simulink model "ofdm_txrx_alamouti"  (Simulink 6.3)
settings: Solver=ode45  StartTime=0.0  StopTime=45000  RelTol=1e-3
InitFcn:
  | ofdm_rx_alamouti_init
StartFcn:
  | ofdm_rx_alamouti_init
values from neighbouring files (symbols this model uses):
  CPLength = 16    [ofdm_tx_alamouti_init.m]
  CRCPolynomial = hex2dec('04c11db7')    [calcTxCRC.m]    (also in ofdm_tx_alamouti_init.m)
  hi = zeros(1,length(cci))    [ofdm_rx_alamouti_init.m]
  hr = zeros(1,length(ccr))    [ofdm_rx_alamouti_init.m]
  max_CP_length = 16    [ofdm_tx_alamouti_init.m]
  max_num_baseRateSymbols = 31    [ofdm_tx_alamouti_init.m]
  max_num_subcarriers = 64    [ofdm_tx_alamouti_init.m]
  max_num_trainingSymbols = 15    [ofdm_tx_alamouti_init.m]
  max_OFDM_symbols = 2047    [ofdm_tx_alamouti_init.m]
  modConstellation_qam16 = 0.75*[1 3 -1 -3]./3    [ofdm_tx_alamouti_init.m]
  modConstellation_qam256 = 0.9375*[3 1 5 7 11 9 13 15 -3 -1 -5 -7 -11 -9 -13 -15]./15    [ofdm_tx_alamouti_init.m]
  modConstellation_qam64 = 0.875*[3 1 5 7 -3 -1 -5 -7]./7    [ofdm_tx_alamouti_init.m]
  modConstellation_qpsk = [1 -1]./sqrt(2)    [ofdm_tx_alamouti_init.m]
  numBaseRateSymbols = 2    [ofdm_tx_alamouti_init.m]
  numFullRateSymbols = numFullRateSymbols + mod(numFullRateSymbols, 2)    [ofdm_tx_alamouti_init.m]
  numSubcarriers = 64    [ofdm_tx_alamouti_init.m]
  numTrainingSymbols = 4    [ofdm_tx_alamouti_init.m]
  pkt_crossCorr_thresh = 0.7    [ofdm_rx_alamouti_init.m]
  pkt_energy_thresh = 0    [ofdm_rx_alamouti_init.m]
  pkt_numPayloadBytes = 24+4+20+20+500    (= 568)    [ofdm_tx_alamouti_init.m]
  pktTiming_count_payloadStart = pktTiming_count_lsStart + pktTiming_ls_num*pktTiming_ls_length    [ofdm_rx_init_packetTimingControl.m]
  preamble = 6*[0 shortsyms_10 longsyms_2]    [ofdm_tx_alamouti_init.m]
  preamble_I = real(preamble)    [ofdm_tx_alamouti_init.m]
  preamble_Q = imag(preamble)    [ofdm_tx_alamouti_init.m]
  RAM_init_size = 4096    [ofdm_tx_alamouti_init.m]
  rx_longCorrCountLoad = 251-16    (= 235)    [ofdm_rx_alamouti_init.m]
  rx_longCorrThresh = 3000    [ofdm_rx_alamouti_init.m]
  RxDataScrambling_Seq = [40 198 78 63 82 173 102 245 48 111 172 115 147 230 216 93 72 65 62 2 205 242 122 90 128 83 105 97 73 10 5 252]    [ofdm_tx_alamouti_init.m]
  simOnly_numSamples = length(preamble)+( (numSubcarriers+CPLength)*(numTrainingSymbols + numBaseRateSymbols + numFullRateSymbols) )    [ofdm_tx_alamouti_init.m]
  train = [train train]    [ofdm_tx_alamouti_init.m]
  TxDataScrambling_Seq = [40 198 78 63 82 173 102 245 48 111 172 115 147 230 216 93 72 65 62 2 205 242 122 90 128 83 105 97 73 10 5 252]    [ofdm_tx_alamouti_init.m]
undefined symbols (not defined in the file or in its folder): CRC_table_gen, gcbh, interpFilter_4, xlBlockLoadCallback
libraries: xbsIndex_r4 x4373, dspmlti4 x4, dspsigops x4, commchan3 x2, simulink x2, commrflib2 x2, commsink2 x2, dspsrcs4 x1
(part 5 of 39)

== system ofdm_txrx_alamouti/Registers/Tx Slices & Gotos ==
  note: LSB
  note: 0x1
  note: 0x2
  note: 0x4
  note: 0x8
  note: 0xF0
  note: 0x100
  note: 0xFFFF0000
  note: 0x200
  note: 0x400
  note: 0x800
  32b : Inport
  Slice1 : xbsIndex_r4/Slice  nbits=1  boolean_output=off  mode=Lower Bit Location + Width  bit1=0  base1=MSB of Input  bit0=10  base0=LSB of Input  dbl_ovrd=off  block_type=slice
      settings: infoedit="Extracts a given range of bits from each input sample and presents it at the output.  The output type is ordinarily unsigned with binary point at zero, but can be Boolean when the slice is one bit wide.<P><P>Hardware notes: In hardware this block costs nothing."  block_version=VER_STRING_GOES_HERE
  Slice14 : xbsIndex_r4/Slice  nbits=1  boolean_output=on  mode=Lower Bit Location + Width  bit1=0  base1=MSB of Input  bit0=0  base0=LSB of Input  dbl_ovrd=off  block_type=slice
      settings: infoedit="Extracts a given range of bits from each input sample and presents it at the output.  The output type is ordinarily unsigned with binary point at zero, but can be Boolean when the slice is one bit wide.<P><P>Hardware notes: In hardware this block costs nothing."  block_version=VER_STRING_GOES_HERE
  Slice18 : xbsIndex_r4/Slice  nbits=1  boolean_output=on  mode=Lower Bit Location + Width  bit1=0  base1=MSB of Input  bit0=1  base0=LSB of Input  dbl_ovrd=off  block_type=slice
      settings: infoedit="Extracts a given range of bits from each input sample and presents it at the output.  The output type is ordinarily unsigned with binary point at zero, but can be Boolean when the slice is one bit wide.<P><P>Hardware notes: In hardware this block costs nothing."  block_version=VER_STRING_GOES_HERE
  Slice19 : xbsIndex_r4/Slice  nbits=1  boolean_output=on  mode=Lower Bit Location + Width  bit1=0  base1=MSB of Input  bit0=2  base0=LSB of Input  dbl_ovrd=off  block_type=slice
      settings: infoedit="Extracts a given range of bits from each input sample and presents it at the output.  The output type is ordinarily unsigned with binary point at zero, but can be Boolean when the slice is one bit wide.<P><P>Hardware notes: In hardware this block costs nothing."  block_version=VER_STRING_GOES_HERE
  Slice2 : xbsIndex_r4/Slice  nbits=1  boolean_output=off  mode=Lower Bit Location + Width  bit1=0  base1=MSB of Input  bit0=11  base0=LSB of Input  dbl_ovrd=off  block_type=slice
      settings: infoedit="Extracts a given range of bits from each input sample and presents it at the output.  The output type is ordinarily unsigned with binary point at zero, but can be Boolean when the slice is one bit wide.<P><P>Hardware notes: In hardware this block costs nothing."  block_version=VER_STRING_GOES_HERE
  Slice20 : xbsIndex_r4/Slice  nbits=1  boolean_output=on  mode=Lower Bit Location + Width  bit1=0  base1=MSB of Input  bit0=3  base0=LSB of Input  dbl_ovrd=off  block_type=slice
      settings: infoedit="Extracts a given range of bits from each input sample and presents it at the output.  The output type is ordinarily unsigned with binary point at zero, but can be Boolean when the slice is one bit wide.<P><P>Hardware notes: In hardware this block costs nothing."  block_version=VER_STRING_GOES_HERE
  Slice21 : xbsIndex_r4/Slice  nbits=4  boolean_output=off  mode=Lower Bit Location + Width  bit1=0  base1=MSB of Input  bit0=4  base0=LSB of Input  dbl_ovrd=off  block_type=slice
      settings: infoedit="Extracts a given range of bits from each input sample and presents it at the output.  The output type is ordinarily unsigned with binary point at zero, but can be Boolean when the slice is one bit wide.<P><P>Hardware notes: In hardware this block costs nothing."  block_version=VER_STRING_GOES_HERE
  Slice27 : xbsIndex_r4/Slice  nbits=16  boolean_output=off  mode=Lower Bit Location + Width  bit1=0  base1=MSB of Input  bit0=16  base0=LSB of Input  dbl_ovrd=off  block_type=slice
      settings: infoedit="Extracts a given range of bits from each input sample and presents it at the output.  The output type is ordinarily unsigned with binary point at zero, but can be Boolean when the slice is one bit wide.<P><P>Hardware notes: In hardware this block costs nothing."  block_version=VER_STRING_GOES_HERE
  Slice28 : xbsIndex_r4/Slice  nbits=1  boolean_output=off  mode=Lower Bit Location + Width  bit1=0  base1=MSB of Input  bit0=8  base0=LSB of Input  dbl_ovrd=off  block_type=slice
      settings: infoedit="Extracts a given range of bits from each input sample and presents it at the output.  The output type is ordinarily unsigned with binary point at zero, but can be Boolean when the slice is one bit wide.<P><P>Hardware notes: In hardware this block costs nothing."  block_version=VER_STRING_GOES_HERE
  Slice29 : xbsIndex_r4/Slice  nbits=1  boolean_output=off  mode=Lower Bit Location + Width  bit1=0  base1=MSB of Input  bit0=9  base0=LSB of Input  dbl_ovrd=off  block_type=slice
      settings: infoedit="Extracts a given range of bits from each input sample and presents it at the output.  The output type is ordinarily unsigned with binary point at zero, but can be Boolean when the slice is one bit wide.<P><P>Hardware notes: In hardware this block costs nothing."  block_version=VER_STRING_GOES_HERE
  tag scope: TxReg_2ndTxAntB=global, TxReg_2ndTxDelay=global, TxReg_2ndTxEn=global, TxReg_AntBPreambleShift=global, TxReg_DisableAntBPreamble=global, TxReg_EnableTxPreCFO=global, TxReg_PilotScrambling=global, TxReg_RelayTxEn=global
  wires:
  32b/1 -> Slice1/1, Slice27/1, Slice2/1, Slice29/1, Slice28/1, Slice21/1, Slice20/1, Slice19/1, Slice18/1, Slice14/1
  Slice19/1 -> [TxReg_DisableAntBPreamble]
  Slice20/1 -> [TxReg_PilotScrambling]
  Slice21/1 -> [TxReg_AntBPreambleShift]
  Slice27/1 -> [TxReg_2ndTxDelay]
  Slice28/1 -> [TxReg_2ndTxEn]
  Slice29/1 -> [TxReg_2ndTxAntB]
  Slice1/1 -> [TxReg_EnableTxPreCFO]
  Slice2/1 -> [TxReg_RelayTxEn]

== system ofdm_txrx_alamouti/OFDM Rx Alamouti/ADCs & Ant Selection/Antenna Selection ==
  AntA_I : Inport
  AntA_Q : Inport  Port=2
  AntB_I : Inport  Port=3
  AntB_Q : Inport  Port=4
  PktDetReset : Inport  Port=5
  Ant Sel Logic : Subsystem
  AntA_Gains : Subsystem
  AntB_Gains : Subsystem
  Concat2 : xbsIndex_r4/Concat  num_inputs=3  dbl_ovrd=off  block_type=concat
      settings: block_version=VER_STRING_GOES_HERE
  Constant6 : xbsIndex_r4/Constant  arith_type=Unsigned  const=0  n_bits=1  bin_pt=0  explicit_period=off  period=1  equ=P=C  opselect=C  inp2=PCIN>>17  opr=+  inp1=P  carry=CIN  dbl_ovrd=off  block_type=constant
      settings: block_version=VER_STRING_GOES_HERE
  Mux : xbsIndex_r4/Mux  inputs=2  en=off  latency=1  precision=Full  arith_type=Unsigned  n_bits=16  bin_pt=14  quantization=Truncate  overflow=Wrap  dbl_ovrd=off  block_type=mux
      settings: block_version=8.2.02
  Mux1 : xbsIndex_r4/Mux  inputs=2  en=off  latency=1  precision=Full  arith_type=Unsigned  n_bits=16  bin_pt=14  quantization=Truncate  overflow=Wrap  dbl_ovrd=off  block_type=mux
      settings: block_version=8.2.02
  Posedge2 : Subsystem
  Register4 : S-Function  LoadFcn=xlBlockLoadCallback(gcbh);  FunctionName=sysgen  Parameters=ptable_  mask(Xilinx Register Block){init=0, rst_sgadvanced=, rst=off, en_sgadvanced=, en=off, dbl_ovrd_sgadvanced=, dbl_ovrd=off, xl_use_area_sgadvanced=, xl_use_area=off, xl_area=[7 14 0 0 0 0 0], has_advanced_control=0, sggui_pos=-1,-1,-1,-1, block_type=register, block_version=VER_STRING_GOES_HERE, sg_icon_stat=30,28,1,1,white,blue,0,ac6b57db, sg_mask_display=|, sg_list_contents=, sg_blockgui_xml=|}
      mask sg_mask_display=|
      | fprintf('','COMMENT: begin icon graphics ');
      | patch([0 30 30 0 ],[0 0 28 28 ],[0.77 0.82 0.91]);
      | patch([8 3 10 3 8 15 17 19 27 21 15 11 19 11 15 21 27 19 17 15 8 ],[3 8 15 22 27 27 25 27 27 21 27 23 15 7 3 9 3 3 5 3 3 ],[0.98 0.96 0.92]);
      | plot([0 0 30 30 0 ],[0 28 28 0 0 ]);
      | fprintf('','COMMENT: end icon graphics');
      |
      | fprintf('','COMMENT: begin icon text ');
      | color('black');port_label('input',1,'d');
      | color('black');port_label('output',1,'q');
      | color('black');disp('\bf{z^{-1}}','texmode','on');
      | fprintf('','COMMENT: end icon text');
      mask sg_blockgui_xml=|
      | <!--   *  Copyright (c) 2005, Xilinx, Inc.  All Rights Reserved.           --><!--   *  Reproduction or reuse, in any form, without the explicit written --><!--   *  consent of Xilinx, Inc., is strictly prohibited.                 --><sysgenblock block_type="register" simulinkname="Register" >
      |  <initialization file="xlregister_init.m" />
      |  <icon width="60" bg_color="blue" height="56" wmark_color="white" />
      |  <dll entry_point="Register_config" name="Sysgen" />
      |  <handlers enablement="xlmagicenablement" />
      |  <libraries>
      |   <library name="xbsIndex" />
      |   <library name="xbsBasic" />
      |   <library name="xbsControl" />
      |   <library name="xbsMemory" />
      |  </libraries>
      |  <blockgui label="Xilinx Register" >
      |   <tabpane>
      |    <tab name="basictab" label="Basic" >
      |     <editbox ctype="Double" evaluate="true" name="init" label="Initial value" default="0" />
      |     <etch label="Optional Ports" >
      |      <checkbox ctype="Int" evaluate="true" name="rst" label="Provide synchronous reset port" default="off" />
      |      <checkbox ctype="Int" evaluate="true" name="en" label="Provide enable port" default="off" />
      |     </etch>
      |    </tab>
      |    <tab name="advancedtab" label="Advanced" >
      |     <etch name="simetch" label="Simulation" >
      |      <checkbox ctype="Int" evaluate="true" name="dbl_ovrd" label="Override with doubles" default="off" />
      |     </etch>
      |    </tab>
      |    <tab name="impltab" label="Implementation" >
      |     <etch label="FPGA Area Estimation" >
      |      <checkbox name="xl_use_area" label="Define FPGA area for resource estimation" default="off" />
      |      <editbox top_label="true" name="xl_area" label="FPGA area [slices, FFs, BRAMs, LUTs, IOBs, emb. mults, TBUFs]" default="[0,0,0,0,0,0,0]" />
      |     </etch>
      |    </tab>
      |   </tabpane>
      |  </blockgui>
      | </sysgenblock>
      mask prompts: init "Initial value"; rst_sgadvanced ""; rst "Provide synchronous reset port"; en_sgadvanced ""; en "Provide enable port"; dbl_ovrd_sgadvanced ""; dbl_ovrd "Override with doubles"; xl_use_area_sgadvanced ""; xl_use_area "Define FPGA area for resource estimation"; xl_area "FPGA area [slices, FFs, BRAMs, LUTs, IOBs, emb. mults, TBUFs]"; has_advanced_control ""; sggui_pos ""; block_type ""; block_version ""; sg_icon_stat ""; sg_mask_display ""; sg_list_contents ""; sg_blockgui_xml ""
      mask help: Xilinx Register
      mask init:
      | try
      |   tmp_gcb = gcb;
      |   tmp_gcbh = gcbh;
      |   xlMungeMaskParams;
      |   block_config = 'Sysgen:Register_config';
      |   serialized_declarations = '{''dbl_ovrd''=>''Int'',''en''=>''Int'',''init''=>''Double'',''rst''=>''Int''}';
      |   xlregister_init();
      |   ptable_ = xlblockprep(get_param(tmp_gcb, 'MaskWSVariables'));
      | catch
      |   global xl_report_errors;
      |   if(~isempty(xl_report_errors) && xl_report_errors)
      |     e = regexprep(lasterr, '\n', '\nError: ');
      |     disp(['Error: While running MaskInit code on block ' tmp_gcb ': ' e]);
      |     error(e);
      |   end
      | end
      settings: CopyFcn=xlBlockMoveCallback(gcbh);  DeleteFcn=xlDestroyGui(gcbh);  ModelCloseFcn=xlDestroyGui(gcbh);  PreSaveFcn=xlBlockPreSaveCallback(gcbh);  PostSaveFcn=xlBlockPostSaveCallback(gcbh);  DestroyFcn=xlDestroyGui(gcbh);  OpenFcn=xlOpenGui(gcbh, @xlmagicenablement, -1)  CloseFcn=xlDestroyGui(gcbh);  MoveFcn=xlBlockMoveCallback(gcbh);
      mask Help: eval('xlWeb(xlhtmldoclink(''Register''))');
      mask Display:
      | fprintf('','COMMENT: begin icon graphics ');
      | patch([0 30 30 0 ],[0 0 28 28 ],[0.77 0.82 0.91]);
      | patch([8 3 10 3 8 15 17 19 27 21 15 11 19 11 15 21 27 19 17 15 8 ],[3 8 15 22 27 27 25 27 27 21 27 23 15 7 3 9 3 3 5 3 3 ],[0.98 0.96 0.92]);
      | plot([0 0 30 30 0 ],[0 28 28 0 0 ]);
      | fprintf('','COMMENT: end icon graphics');
      | 
      | fprintf('','COMMENT: begin icon text ');
      | color('black');port_label('input',1,'d');
      | color('black');port_label('output',1,'q');
      | color('black');disp('\bf{z^{-1}}','texmode','on');
      | fprintf('','COMMENT: end icon text');
  Relational : xbsIndex_r4/Relational  mode=a>b  en=off  latency=0  dbl_ovrd=off  block_type=relational
      settings: block_version=8.2.02
  Rx_Gains : xbsIndex_r4/To Register  shared_memory_name='Rx_Gains'  init=0  ownership=Locally owned and initialized  explicit_data_type=off  arith_type="Signed  (2's comp)"  n_bits=16  bin_pt=14  dbl_ovrd=off  block_type=toreg
      settings: AttributesFormatString=<< %<shared_memory_name> >>  infoedit="Register block that writes data to a shared memory register.  Delay of one sample period."  block_version=VER_STRING_GOES_HERE
  Rx_debug_antSel : xbsIndex_r4/Gateway Out  hdl_port=on  timing_constraint=None  locs_specified=off  LOCs={}  block_type=gatewayout
      settings: infoedit="Gateway out block.  Converts Xilinx fixed point inputs into ouputs of type Simulink integer, double, or fixed point.<P><P>Hardware notes:  In hardware these blocks become top level output ports or are discarded, depending on how they are configured."  xl_area=[0 0 0 0 1 0 0]  block_version=VER_STRING_GOES_HERE
  Terminator : Terminator
  Up Sample : xbsIndex_r4/Up Sample  sample_ratio=4  copy_samples=on  dbl_ovrd=off  block_type=usamp
      settings: infoedit="Up samples input data.  Inserted values  can be zeros or copies of the most recent input sample.<P><P>Hardware notes: No hardware is needed if inserted values are copies of the input sample; otherwise, a mux and single bit flip-flop are used."  block_version=VER_STRING_GOES_HERE
  Up Sample1 : xbsIndex_r4/Up Sample  sample_ratio=4  copy_samples=on  dbl_ovrd=off  block_type=usamp
      settings: infoedit="Up samples input data.  Inserted values  can be zeros or copies of the most recent input sample.<P><P>Hardware notes: No hardware is needed if inserted values are copies of the input sample; otherwise, a mux and single bit flip-flop are used."  block_version=VER_STRING_GOES_HERE
  Up Sample2 : xbsIndex_r4/Up Sample  sample_ratio=4  copy_samples=on  dbl_ovrd=off  block_type=usamp
      settings: infoedit="Up samples input data.  Inserted values  can be zeros or copies of the most recent input sample.<P><P>Hardware notes: No hardware is needed if inserted values are copies of the input sample; otherwise, a mux and single bit flip-flop are used."  block_version=VER_STRING_GOES_HERE
  I : Outport
  Q : Outport  Port=2
  wires:
  AntB_I/1 -> Mux/3
  AntB_Q/1 -> Mux1/3
  AntA_I/1 -> Mux/2
  AntA_Q/1 -> Mux1/2
  Mux/1 -> I/1
  Mux1/1 -> Q/1
  PktDetReset/1 -> Up Sample1/1, AntB_Gains/1(Rst), AntA_Gains/1(Rst)
  AntA_Gains/2(GainVec) -> Relational/1, Concat2/3
  AntB_Gains/2(GainVec) -> Relational/2, Concat2/1
  Relational/1 -> Ant Sel Logic/1(G(B) < G(A))
  Ant Sel Logic/1(AntSel) -> Mux/1, Mux1/1, Up Sample2/1
  Up Sample2/1 -> Register4/1
  AntB_Gains/1(Vout) -> Ant Sel Logic/2(B_Vin)
  Concat2/1 -> Up Sample/1
  Up Sample/1 -> Rx_Gains/1
  Posedge2/1(Q) -> Rx_Gains/2
  Constant6/1 -> Concat2/2
  Register4/1 -> Rx_debug_antSel/1
  Up Sample1/1 -> Posedge2/1(D)
  Rx_Gains/1 -> Terminator/1

== system ofdm_txrx_alamouti/OFDM Rx Alamouti/Coarse Freq Correction1/A*B ==
  Re[A] : Inport
  Im[A] : Inport  Port=2
  Im[B] : Inport  Port=3
  Re[B] : Inport  Port=4
  Add1 : xbsIndex_r4/AddSub  mode=Addition  use_carryin=off  use_carryout=off  en=off  latency=0  precision=User Defined  arith_type="Signed  (2's comp)"  n_bits=17  bin_pt=15  quantization=Truncate  overflow=Flag as error  dbl_ovrd=off  use_behavioral_HDL=on  pipelined=off  use_rpm=on  block_type=addsub
      settings: xl_area=[9 0 0 16 0 0 0]  block_version=VER_STRING_GOES_HERE
  Counter : xbsIndex_r4/Counter  Orientation=down  cnt_type=Free Running  cnt_to=4  operation=Up  start_count=0  cnt_by_val=1  arith_type=Unsigned  n_bits=2  bin_pt=0  load_pin=off  rst=off  en=off  explicit_period=on  period=1  dbl_ovrd=off  use_behavioral_HDL=off  use_rpm=on  block_type=counter
      settings: xl_area=[3 3 0 4 0 0 0]  block_version=VER_STRING_GOES_HERE
  Delay1 : xbsIndex_r4/Delay  en=off  latency=1  dbl_ovrd=off  reg_retiming=off  block_type=delay
      settings: infoedit=Hardware notes: A delay line is a chain, each link of which is an SRL16 followed by a flip-flop. If register retiming is enabled, the delay line is a chain of flip-flops.  xl_area=[8 16 0 0 0 0 0]  block_version=VER_STRING_GOES_HERE
  Delay2 : xbsIndex_r4/Delay  en=off  latency=2  dbl_ovrd=off  reg_retiming=off  block_type=delay
      settings: infoedit=Hardware notes: A delay line is a chain, each link of which is an SRL16 followed by a flip-flop. If register retiming is enabled, the delay line is a chain of flip-flops.  xl_area=[9 17 0 17 0 0 0]  block_version=VER_STRING_GOES_HERE
  Down Sample3 : xbsIndex_r4/Down Sample  sample_ratio=4  sample_phase=First Value of Frame  en=off  latency=1  dbl_ovrd=off  block_type=dsamp
      settings: xl_area=[9 17 0 0 0 0 0]  block_version=VER_STRING_GOES_HERE
  Down Sample4 : xbsIndex_r4/Down Sample  sample_ratio=4  sample_phase=First Value of Frame  en=off  latency=1  dbl_ovrd=off  block_type=dsamp
      settings: xl_area=[9 17 0 0 0 0 0]  block_version=VER_STRING_GOES_HERE
  Mult4 : xbsIndex_r4/Mult  precision=User Defined  arith_type="Signed  (2's comp)"  n_bits=17  bin_pt=16  quantization=Truncate  overflow=Flag as error  en=off  latency=1  dbl_ovrd=off  use_behavioral_HDL=off  use_embedded=on  opt=Speed  optimum_pipeline=off  use_rpm=on  placement_style=Rectangular shape  pipeline=off  block_type=mult
      settings: infoedit=Hardware notes: To use the internal pipeline stage of the dedicated multiplier you must select 'Pipeline for maximum performance'.  xl_area=[14 27 0 27 0 1 0]  block_version=VER_STRING_GOES_HERE
  Mux : xbsIndex_r4/Mux  inputs=4  en=off  latency=0  precision=Full  arith_type="Signed  (2's comp)"  n_bits=16  bin_pt=13  quantization=Truncate  overflow=Wrap  dbl_ovrd=off  block_type=mux
      settings: xl_area=[14 0 0 28 0 0 0]  block_version=VER_STRING_GOES_HERE
  Mux1 : xbsIndex_r4/Mux  inputs=4  en=off  latency=0  precision=Full  arith_type="Signed  (2's comp)"  n_bits=16  bin_pt=13  quantization=Truncate  overflow=Wrap  dbl_ovrd=off  block_type=mux
      settings: xl_area=[14 0 0 28 0 0 0]  block_version=VER_STRING_GOES_HERE
  Negate : xbsIndex_r4/Negate  precision=Full  arith_type="Signed  (2's comp)"  n_bits=14  bin_pt=13  quantization=Truncate  overflow=Flag as error  en=off  latency=0  dbl_ovrd=off  block_type=negate
      settings: xl_area=[7 0 0 14 0 0 0]  block_version=VER_STRING_GOES_HERE
  Slice : xbsIndex_r4/Slice  nbits=2  boolean_output=off  mode=Lower Bit Location + Width  bit1=0  base1=MSB of Input  bit0=0  base0=LSB of Input  dbl_ovrd=off  block_type=slice
      settings: infoedit="Extracts a given range of bits from each input sample and presents it at the output.  The output type is ordinarily unsigned with binary point at zero, but can be Boolean when the slice is one bit wide.<P><P>Hardware notes: In hardware this block costs nothing."  block_version=VER_STRING_GOES_HERE
  Up Sample{1..4} (4 alike) : xbsIndex_r4/Up Sample  sample_ratio=4  copy_samples=on  dbl_ovrd=off  block_type=usamp
      settings: infoedit="Up samples input data.  Inserted values  can be zeros or copies of the most recent input sample.<P><P>Hardware notes: No hardware is needed if inserted values are copies of the input sample; otherwise, a mux and single bit flip-flop are used."  xl_area=[0 0 0 0 0 0 0]  block_version=VER_STRING_GOES_HERE
  Re : Outport
  Im1 : Outport  Port=2
  wires:
  Negate/1 -> Mux1/3
  Add1/1 -> Down Sample4/1, Delay2/1
  Down Sample3/1 -> Re/1
  Down Sample4/1 -> Im1/1
  Delay2/1 -> Down Sample3/1
  Mux/1 -> Mult4/2
  Mux1/1 -> Mult4/1
  Im[B]/1 -> Up Sample4/1
  Im[A]/1 -> Up Sample1/1
  Re[B]/1 -> Up Sample2/1
  Re[A]/1 -> Up Sample3/1
  Up Sample3/1 -> Mux1/2, Mux1/4
  Up Sample2/1 -> Mux/5, Mux/2
  Up Sample4/1 -> Mux/3, Mux/4
  Up Sample1/1 -> Negate/1, Mux1/5
  Mult4/1 -> Add1/1, Delay1/1
  Delay1/1 -> Add1/2
  Counter/1 -> Slice/1
  Slice/1 -> Mux1/1, Mux/1

== system ofdm_txrx_alamouti/OFDM Rx Alamouti/Coarse Freq Correction1/Coarse Freq Offset Calc Combo ==
  I In : Inport
  Q In : Inport  Port=2
  Reset : Inport  Port=3
  PktDet : Inport  Port=4
  Accum : xbsIndex_r4/Accumulator  operation=Add  n_bits=7+13  overflow=Flag as error  scale=1  rst=on  hasbypass=on  en=on  dbl_ovrd=off  use_behavioral_HDL=on  block_type=accum
      settings: infoedit="Adder or subtractor-based accumulator.   Output type and binary point position match the input.<P><P>Hardware notes: When \"Reinitialize with input 'b' on reset\" is selected, the accumulator is forced to run at the system rate even if the input 'b' is running at a slower rate."  xl_area=[17 33 0 32 0 0 0]  block_version=VER_STRING_GOES_HERE
  Accum Control : Subsystem
  Arctan2 : Subsystem
  Coarse Freq Scope1 : Scope  NumInputPorts=5
      settings: TimeRange=3500  YMin=-3.5~0~0~0~0  YMax=3.5~1~75~0.00035~0.000275  SaveName=ScopeData39
  Coarse Freq Scope4 : Scope  NumInputPorts=5
      settings: TimeRange=3500  YMin=-5~-0.0001~0~0~0  YMax=12.5~0.00035~0.00035~1~1  SaveName=ScopeData43
  Convert : xbsIndex_r4/Convert  arith_type=Unsigned  n_bits=32  bin_pt=32  quantization=Truncate  overflow=Wrap  latency=0  dbl_ovrd=off  pipeline=off  block_type=convert
      settings: xl_area=[0 0 0 0 0 0 0]  block_version=VER_STRING_GOES_HERE
  Convert1 : xbsIndex_r4/Convert  arith_type=Unsigned  n_bits=32  bin_pt=32  quantization=Truncate  overflow=Wrap  latency=0  dbl_ovrd=off  pipeline=off  block_type=convert
      settings: xl_area=[0 0 0 0 0 0 0]  block_version=VER_STRING_GOES_HERE
  Counter : xbsIndex_r4/Counter  cnt_type=Free Running  cnt_to=Inf  operation=Up  start_count=0  cnt_by_val=1  arith_type=Unsigned  n_bits=7  bin_pt=0  load_pin=off  rst=on  en=on  explicit_period=off  period=1  dbl_ovrd=off  use_behavioral_HDL=off  use_rpm=on  block_type=counter
      settings: xl_area=[6 11 0 12 0 0 0]  block_version=VER_STRING_GOES_HERE
  Delay1 : xbsIndex_r4/Delay  en=off  latency=4  dbl_ovrd=off  reg_retiming=off  block_type=delay
      settings: infoedit=Hardware notes: A delay line is a chain, each link of which is an SRL16 followed by a flip-flop. If register retiming is enabled, the delay line is a chain of flip-flops.  block_version=VER_STRING_GOES_HERE
  Delay4 : xbsIndex_r4/Delay  en=off  latency=4  dbl_ovrd=off  reg_retiming=off  block_type=delay
      settings: infoedit=Hardware notes: A delay line is a chain, each link of which is an SRL16 followed by a flip-flop. If register retiming is enabled, the delay line is a chain of flip-flops.  block_version=VER_STRING_GOES_HERE
  Delay5 : xbsIndex_r4/Delay  en=off  latency=1  dbl_ovrd=off  reg_retiming=off  block_type=delay
      settings: infoedit=Hardware notes: A delay line is a chain, each link of which is an SRL16 followed by a flip-flop. If register retiming is enabled, the delay line is a chain of flip-flops.  block_version=VER_STRING_GOES_HERE
  Delay6 : xbsIndex_r4/Delay  en=off  latency=2  dbl_ovrd=off  reg_retiming=off  block_type=delay
      settings: infoedit=Hardware notes: A delay line is a chain, each link of which is an SRL16 followed by a flip-flop. If register retiming is enabled, the delay line is a chain of flip-flops.  block_version=VER_STRING_GOES_HERE
  Gateway Out{1..5,19..23} (10 alike) : xbsIndex_r4/Gateway Out  hdl_port=off  timing_constraint=None  locs_specified=off  LOCs={}  block_type=gatewayout
      settings: infoedit="Gateway out block.  Converts Xilinx fixed point inputs into ouputs of type Simulink integer, double, or fixed point.<P><P>Hardware notes:  In hardware these blocks become top level output ports or are discarded, depending on how they are configured."  xl_area=[0 0 0 0 0 0 0]  block_version=VER_STRING_GOES_HERE
  Inverter1 : xbsIndex_r4/Inverter  en=off  latency=0  dbl_ovrd=off  block_type=inv
      settings: xl_area=[0 0 0 0 0 0 0]  block_version=VER_STRING_GOES_HERE
  Inverter2 : xbsIndex_r4/Inverter  en=off  latency=0  dbl_ovrd=off  block_type=inv
      settings: xl_area=[0 0 0 0 0 0 0]  block_version=VER_STRING_GOES_HERE
  Mult1 : xbsIndex_r4/Mult  precision=User Defined  arith_type="Signed  (2's comp)"  n_bits=35  bin_pt=33  quantization="Round  (unbiased: +/- Inf)"  overflow=Flag as error  en=off  latency=2  dbl_ovrd=off  use_behavioral_HDL=off  use_embedded=on  opt=Speed  optimum_pipeline=off  use_rpm=off  placement_style=Rectangular shape  pipeline=off  block_type=mult
      settings: infoedit=Hardware notes: To use the internal pipeline stage of the dedicated multiplier you must select 'Pipeline for maximum performance'.  xl_area=[52 69 0 102 0 2 0]  block_version=VER_STRING_GOES_HERE
  Mux2 : xbsIndex_r4/Mux  inputs=2  en=off  latency=0  precision=Full  arith_type="Signed  (2's comp)"  n_bits=8  bin_pt=2  quantization=Truncate  overflow=Wrap  dbl_ovrd=off  block_type=mux
      settings: xl_area=[0, 0, 0, 0, 0, 0, 0]  block_version=VER_STRING_GOES_HERE
  Phase Noise Tracker1 : Subsystem
  PhaseDiff : Subsystem
  Posedge : Subsystem
  Posedge1 : Subsystem
  Register1 : xbsIndex_r4/Register  init=0  rst=on  en=on  dbl_ovrd=off  block_type=register
      settings: block_version=8.2.01
  Register4 : xbsIndex_r4/Register  init=0  rst=on  en=on  dbl_ovrd=off  block_type=register
      settings: block_version=8.2.01
  inv 2pi : xbsIndex_r4/Constant  arith_type=Unsigned  const=1/(2*pi*64*72)  n_bits=24  bin_pt=24  explicit_period=off  period=1  equ=P=C  opselect=C  inp2=PCIN>>17  opr=+  inp1=P  carry=CIN  dbl_ovrd=off  block_type=constant
      settings: block_version=VER_STRING_GOES_HERE
  Phase Inc : Outport
  wires:
  PktDet/1 -> Accum Control/1(En)
  Q In/1 -> Arctan2/2(Q)
  I In/1 -> Arctan2/1(I)
  Accum Control/1(" En") -> Delay4/1
  Gateway Out19/1 -> Coarse Freq Scope4/1
  Gateway Out20/1 -> Coarse Freq Scope4/2
  Gateway Out21/1 -> Coarse Freq Scope4/3
  Gateway Out22/1 -> Coarse Freq Scope4/4
  Gateway Out23/1 -> Coarse Freq Scope4/5
  PhaseDiff/1(diff(p0,p63)) -> Accum/1, Gateway Out1/1, Phase Noise Tracker1/1(Phase Err)
  Inverter1/1 -> Accum/2, Posedge/1(D)
  Delay4/1 -> Delay5/1
  Accum/1 -> Mult1/1, Gateway Out22/1
  inv 2pi/1 -> Mult1/2
  Mult1/1 -> Register4/1, Gateway Out21/1
  Posedge/1(Q) -> Delay6/1
  Delay6/1 -> Register4/3, Gateway Out20/1
  Register4/1 -> Gateway Out19/1, Gateway Out5/1, Convert1/1
  Reset/1 -> Accum Control/2(Reset), Register4/2, Counter/1, Phase Noise Tracker1/2(Reset), Register1/2, Gateway Out23/1
  Arctan2/1(atan) -> PhaseDiff/1(p)
  Delay5/1 -> Inverter1/1, Accum/3, Counter/2, Gateway Out2/1, Phase Noise Tracker1/3(Vin), Inverter2/1
  Counter/1 -> Gateway Out3/1, Phase Noise Tracker1/4(Samp Index)
  Gateway Out1/1 -> Coarse Freq Scope1/1
  Gateway Out2/1 -> Coarse Freq Scope1/2
  Gateway Out3/1 -> Coarse Freq Scope1/3
  Gateway Out4/1 -> Coarse Freq Scope1/4
  Gateway Out5/1 -> Coarse Freq Scope1/5
  Phase Noise Tracker1/1(theta) -> Gateway Out4/1, Register1/1
  Convert/1 -> Mux2/3
  Register1/1 -> Convert/1
  Delay1/1 -> Register1/3
  Inverter2/1 -> Posedge1/1(D)
  Posedge1/1(Q) -> Delay1/1
  Convert1/1 -> Mux2/2
  [regRx_newCoarseCFO] -> Mux2/1
  Mux2/1 -> Phase Inc/1

== system ofdm_txrx_alamouti/OFDM Rx Alamouti/Coarse Freq Correction1/DDS ==
  PhaseInc : Inport
  Rst : Inport  Port=2
  "DDS v4_0 " : xbsIndex_r4/DDS v4_0   Description=Direct Digital Synthesizer  mode=Sine and Cosine  neg_sin=on  neg_cos=off  n_bits=24  phase_increment_type=Register  phase_increment=1/10  phase_offset_type=Constant  phase_offset=0  rst=on  en=off  lut_addr_bits=10  acc_latency=One Cycle  acc_n_bits=32  phase_dithering=off  dbl_ovrd=off  explicit_period=off  period=1  mem_type=Block RAM  pipeline=off  use_rpm=off  block_type=ddsv4
      settings: block_version=VER_STRING_GOES_HERE
  Logical1 : xbsIndex_r4/Logical  logical_function=OR  inputs=2  en=off  latency=0  precision=Full  arith_type=Unsigned  n_bits=8  bin_pt=2  align_bp=on  dbl_ovrd=off  block_type=logical
      settings: xl_area=[1 0 0 1 0 0 0]  block_version=VER_STRING_GOES_HERE
  inv 2pi2 : xbsIndex_r4/Constant  arith_type=Boolean  const=1  n_bits=32  bin_pt=32  explicit_period=off  period=1  equ=P=C  opselect=C  inp2=PCIN>>17  opr=+  inp1=P  carry=CIN  dbl_ovrd=off  block_type=constant
      settings: block_version=VER_STRING_GOES_HERE
  Q : Outport
  I : Outport  Port=2
  wires:
  "DDS v4_0 "/2 -> I/1
  "DDS v4_0 "/1 -> Q/1
  Rst/1 -> Logical1/1
  inv 2pi2/1 -> "DDS v4_0 "/2
  PhaseInc/1 -> "DDS v4_0 "/1
  Logical1/1 -> "DDS v4_0 "/3
  [regRx_freqCorrBypass] -> Logical1/2

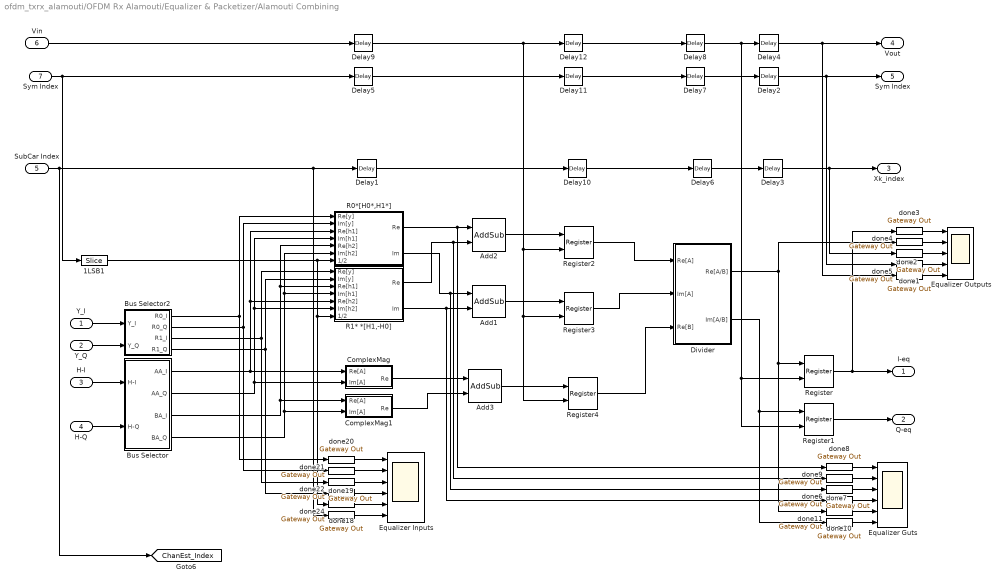
== system ofdm_txrx_alamouti/OFDM Rx Alamouti/Equalizer & Packetizer/Alamouti Combining ==
  Y_I : Inport
  Y_Q : Inport  Port=2
  H-I : Inport  Port=3
  H-Q : Inport  Port=4
  SubCar Index : Inport  Port=5
  Vin : Inport  Port=6
  Sym Index : Inport  Port=7
  1LSB1 : xbsIndex_r4/Slice  nbits=1  boolean_output=off  mode=Lower Bit Location + Width  bit1=0  base1=MSB of Input  bit0=0  base0=LSB of Input  dbl_ovrd=off  block_type=slice
      settings: infoedit="Extracts a given range of bits from each input sample and presents it at the output.  The output type is ordinarily unsigned with binary point at zero, but can be Boolean when the slice is one bit wide.<P><P>Hardware notes: In hardware this block costs nothing."  block_version=VER_STRING_GOES_HERE
  Add1 : xbsIndex_r4/AddSub  mode=Addition  use_carryin=off  use_carryout=off  en=off  latency=0  precision=Full  arith_type="Signed  (2's comp)"  n_bits=18  bin_pt=16  quantization=Truncate  overflow=Flag as error  dbl_ovrd=off  use_behavioral_HDL=on  pipelined=off  use_rpm=on  block_type=addsub
      settings: xl_area=[9 0 0 16 0 0 0]  block_version=VER_STRING_GOES_HERE
  Add2 : xbsIndex_r4/AddSub  mode=Addition  use_carryin=off  use_carryout=off  en=off  latency=0  precision=Full  arith_type="Signed  (2's comp)"  n_bits=18  bin_pt=16  quantization=Truncate  overflow=Flag as error  dbl_ovrd=off  use_behavioral_HDL=on  pipelined=off  use_rpm=on  block_type=addsub
      settings: xl_area=[9 0 0 16 0 0 0]  block_version=VER_STRING_GOES_HERE
  Add3 : xbsIndex_r4/AddSub  mode=Addition  use_carryin=off  use_carryout=off  en=off  latency=0  precision=Full  arith_type="Signed  (2's comp)"  n_bits=18  bin_pt=16  quantization=Truncate  overflow=Flag as error  dbl_ovrd=off  use_behavioral_HDL=on  pipelined=off  use_rpm=on  block_type=addsub
      settings: xl_area=[9 0 0 16 0 0 0]  block_version=VER_STRING_GOES_HERE
  Bus Selector : Subsystem
  Bus Selector2 : Subsystem
  ComplexMag : Subsystem
  ComplexMag1 : Subsystem
  Delay1 : xbsIndex_r4/Delay  en=off  latency=2  dbl_ovrd=off  reg_retiming=off  block_type=delay
      settings: infoedit=Hardware notes: A delay line is a chain, each link of which is an SRL16 followed by a flip-flop. If register retiming is enabled, the delay line is a chain of flip-flops.  xl_area=[0, 0, 0, 0, 0, 0, 0]  block_version=VER_STRING_GOES_HERE
  Delay{2..4,10..12} (6 alike) : xbsIndex_r4/Delay  en=off  latency=1  dbl_ovrd=off  reg_retiming=off  block_type=delay
      settings: infoedit=Hardware notes: A delay line is a chain, each link of which is an SRL16 followed by a flip-flop. If register retiming is enabled, the delay line is a chain of flip-flops.  xl_area=[0, 0, 0, 0, 0, 0, 0]  block_version=VER_STRING_GOES_HERE
  Delay5 : xbsIndex_r4/Delay  en=off  latency=2  dbl_ovrd=off  reg_retiming=off  block_type=delay
      settings: infoedit=Hardware notes: A delay line is a chain, each link of which is an SRL16 followed by a flip-flop. If register retiming is enabled, the delay line is a chain of flip-flops.  xl_area=[0, 0, 0, 0, 0, 0, 0]  block_version=VER_STRING_GOES_HERE
  Delay6 : xbsIndex_r4/Delay  en=off  latency=23-2  dbl_ovrd=off  reg_retiming=off  block_type=delay
      settings: infoedit=Hardware notes: A delay line is a chain, each link of which is an SRL16 followed by a flip-flop. If register retiming is enabled, the delay line is a chain of flip-flops.  xl_area=[0, 0, 0, 0, 0, 0, 0]  block_version=VER_STRING_GOES_HERE
  Delay7 : xbsIndex_r4/Delay  en=off  latency=23-2  dbl_ovrd=off  reg_retiming=off  block_type=delay
      settings: infoedit=Hardware notes: A delay line is a chain, each link of which is an SRL16 followed by a flip-flop. If register retiming is enabled, the delay line is a chain of flip-flops.  xl_area=[0, 0, 0, 0, 0, 0, 0]  block_version=VER_STRING_GOES_HERE
  Delay8 : xbsIndex_r4/Delay  en=off  latency=23-2  dbl_ovrd=off  reg_retiming=off  block_type=delay
      settings: infoedit=Hardware notes: A delay line is a chain, each link of which is an SRL16 followed by a flip-flop. If register retiming is enabled, the delay line is a chain of flip-flops.  xl_area=[0, 0, 0, 0, 0, 0, 0]  block_version=VER_STRING_GOES_HERE
  Delay9 : xbsIndex_r4/Delay  en=off  latency=2  dbl_ovrd=off  reg_retiming=off  block_type=delay
      settings: infoedit=Hardware notes: A delay line is a chain, each link of which is an SRL16 followed by a flip-flop. If register retiming is enabled, the delay line is a chain of flip-flops.  xl_area=[0, 0, 0, 0, 0, 0, 0]  block_version=VER_STRING_GOES_HERE
  Divider : Subsystem
  Equalizer Guts : Scope  NumInputPorts=6
      settings: TimeRange=4000  YMin=-0.125~-0.1~-0.125~-0.125~-1.25~-1  YMax=0.125~0.125~0.125~0.1~1.25~1.25  SaveName=ScopeData38
  Equalizer Outputs : Scope  NumInputPorts=5
      settings: TimeRange=4000  YMin=-0.075~-0.2~0~15~0  YMax=0.075~0.2~70~21~1  SaveName=ScopeData26
  Equalizer Inputs : Scope  NumInputPorts=6
      settings: TimeRange=12000  YMin=-0.5~-0.5~-0.5~-0.5~0~0  YMax=0.5~1~0.5~1~1~100  SaveName=ScopeData18
  R0*[H0*,H1*] : Subsystem
  R1* *[H1,-H0] : Subsystem
  Register{,1..4} (5 alike) : xbsIndex_r4/Register  init=0  rst=off  en=on  dbl_ovrd=off  block_type=register
      settings: block_version=VER_STRING_GOES_HERE
  done{1..11,18..22,24} (17 alike) : xbsIndex_r4/Gateway Out  hdl_port=off  timing_constraint=None  locs_specified=off  LOCs={}  block_type=gatewayout
      settings: infoedit="Gateway out block.  Converts Xilinx fixed point inputs into ouputs of type Simulink integer, double, or fixed point.<P><P>Hardware notes:  In hardware these blocks become top level output ports or are discarded, depending on how they are configured."  xl_area=[0 0 0 0 1 0 0]  block_version=VER_STRING_GOES_HERE
  I-eq : Outport
  Q-eq : Outport  Port=2
  " Xk_index" : Outport  Port=3
  Vout : Outport  Port=4
  " Sym Index" : Outport  Port=5
  tag scope: ChanEst_Index=global
  wires:
  H-I/1 -> Bus Selector/1(H-I)
  H-Q/1 -> Bus Selector/2(H-Q)
  SubCar Index/1 -> [ChanEst_Index], Delay1/1, done24/1
  done18/1 -> Equalizer Inputs/5
  done20/1 -> Equalizer Inputs/1
  done21/1 -> Equalizer Inputs/2
  done19/1 -> Equalizer Inputs/3
  done22/1 -> Equalizer Inputs/4
  done24/1 -> Equalizer Inputs/6
  done3/1 -> Equalizer Outputs/1
  done4/1 -> Equalizer Outputs/2
  done2/1 -> Equalizer Outputs/3
  Vin/1 -> Delay9/1
  done1/1 -> Equalizer Outputs/4
  Sym Index/1 -> Delay5/1, 1LSB1/1
  Y_I/1 -> Bus Selector2/1(Y_I)
  Y_Q/1 -> Bus Selector2/2(Y_Q)
  R1* *[H1,-H0]/1(Re) -> Add2/2, done9/1
  R1* *[H1,-H0]/2(Im) -> Add1/2, done6/1
  Delay1/1 -> Delay10/1
  Delay5/1 -> Delay11/1
  Delay9/1 -> Register2/2, Register3/2, Register4/2, Delay12/1
  done5/1 -> Equalizer Outputs/5
  Register/1 -> I-eq/1, done3/1
  Register1/1 -> Q-eq/1
  1LSB1/1 -> R0*[H0*,H1*]/7(1/2), R1* *[H1,-H0]/7(1/2), done18/1
  R0*[H0*,H1*]/2(Im) -> Add1/1, done7/1
  R0*[H0*,H1*]/1(Re) -> Add2/1, done8/1
  Bus Selector2/1(R0_I) -> R0*[H0*,H1*]/1(Re[y]), done20/1
  Bus Selector2/2(R0_Q) -> R0*[H0*,H1*]/2(Im[y]), done21/1
  Bus Selector2/3(R1_I) -> R1* *[H1,-H0]/1(Re[y]), done19/1
  Bus Selector2/4(R1_Q) -> R1* *[H1,-H0]/2(Im[y]), done22/1
  Bus Selector/1(AA_I) -> R0*[H0*,H1*]/3(Re[h1]), R1* *[H1,-H0]/5(Re[h2]), ComplexMag/1(Re[A])
  Bus Selector/2(AA_Q) -> R0*[H0*,H1*]/4(Im[h1]), R1* *[H1,-H0]/6(Im[h2]), ComplexMag/2(Im[A])
  Bus Selector/3(BA_I) -> R0*[H0*,H1*]/5(Re[h2]), R1* *[H1,-H0]/3(Re[h1]), ComplexMag1/1(Re[A])
  Bus Selector/4(BA_Q) -> R0*[H0*,H1*]/6(Im[h2]), R1* *[H1,-H0]/4(Im[h1]), ComplexMag1/2(Im[A])
  Delay2/1 -> " Sym Index"/1, done1/1
  Delay3/1 -> " Xk_index"/1, done2/1
  Delay4/1 -> Vout/1, done5/1
  done8/1 -> Equalizer Guts/1
  done9/1 -> Equalizer Guts/2
  done7/1 -> Equalizer Guts/3
  done6/1 -> Equalizer Guts/4
  done10/1 -> Equalizer Guts/5
  done11/1 -> Equalizer Guts/6
  ComplexMag/1(Re) -> Add3/1
  ComplexMag1/1(Re) -> Add3/2
  Divider/1(Re[A/B]) -> done4/1, Register/1, done10/1
  Divider/2(Im[A/B]) -> Register1/1, done11/1
  Delay6/1 -> Delay3/1
  Delay7/1 -> Delay2/1
  Delay8/1 -> Register1/2, Register/2, Delay4/1
  Add2/1 -> Register2/1
  Add1/1 -> Register3/1
  Add3/1 -> Register4/1
  Register2/1 -> Divider/1(Re[A])
  Register3/1 -> Divider/2(Im[A])
  Register4/1 -> Divider/3(Re[B])
  Delay10/1 -> Delay6/1
  Delay12/1 -> Delay8/1
  Delay11/1 -> Delay7/1

== system ofdm_txrx_alamouti/OFDM Rx Alamouti/Equalizer & Packetizer/Loop Filter A ==
  Phase Err : Inport
  Rst : Inport  Port=2
  Vin : Inport  Port=3
  Delay1 : xbsIndex_r4/Delay  en=off  latency=3  dbl_ovrd=off  reg_retiming=off  block_type=delay
      settings: infoedit=Hardware notes: A delay line is a chain, each link of which is an SRL16 followed by a flip-flop. If register retiming is enabled, the delay line is a chain of flip-flops.  block_version=VER_STRING_GOES_HERE
  Down Sample1 : xbsIndex_r4/Down Sample  sample_ratio=2  sample_phase="Last Value of Frame  (most efficient)"  en=off  latency=1  dbl_ovrd=off  block_type=dsamp
      settings: block_version=VER_STRING_GOES_HERE
  Gateway Out1 : xbsIndex_r4/Gateway Out  hdl_port=off  timing_constraint=None  locs_specified=off  LOCs={}  block_type=gatewayout
      settings: infoedit="Gateway out block.  Converts Xilinx fixed point inputs into ouputs of type Simulink integer, double, or fixed point.<P><P>Hardware notes:  In hardware these blocks become top level output ports or are discarded, depending on how they are configured."  block_version=VER_STRING_GOES_HERE
  Gateway Out2 : xbsIndex_r4/Gateway Out  hdl_port=off  timing_constraint=None  locs_specified=off  LOCs={}  block_type=gatewayout
      settings: infoedit="Gateway out block.  Converts Xilinx fixed point inputs into ouputs of type Simulink integer, double, or fixed point.<P><P>Hardware notes:  In hardware these blocks become top level output ports or are discarded, depending on how they are configured."  block_version=VER_STRING_GOES_HERE
  Gateway Out6 : xbsIndex_r4/Gateway Out  hdl_port=off  timing_constraint=None  locs_specified=off  LOCs={}  block_type=gatewayout
      settings: infoedit="Gateway out block.  Converts Xilinx fixed point inputs into ouputs of type Simulink integer, double, or fixed point.<P><P>Hardware notes:  In hardware these blocks become top level output ports or are discarded, depending on how they are configured."  block_version=VER_STRING_GOES_HERE
  MIMO CFO Loop Filt : Scope  NumInputPorts=6
      settings: TimeRange=50000  YMin=0~-8.01086e-005~-1~-1~-1~-1  YMax=1~-7.24792e-005~1~1~1~1  SaveName=ScopeData40
  Mult1 : xbsIndex_r4/Mult  precision=User Defined  arith_type="Signed  (2's comp)"  n_bits=32  bin_pt=31  quantization=Truncate  overflow=Flag as error  en=off  latency=3  dbl_ovrd=off  use_behavioral_HDL=off  use_embedded=on  opt=Speed  optimum_pipeline=off  use_rpm=off  placement_style=Rectangular shape  pipeline=on  block_type=mult
      settings: infoedit=Hardware notes: To use the internal pipeline stage of the dedicated multiplier you must select 'Pipeline for maximum performance'.  block_version=VER_STRING_GOES_HERE
  Register1 : xbsIndex_r4/Register  init=0  rst=on  en=on  dbl_ovrd=off  block_type=register
      settings: block_version=8.2.02
  Rx_FreqOffFilt_A_KP : xbsIndex_r4/From Register  shared_memory_name='Rx_FreqOffFilt_A_KP'  init=freqOffset_track_filtCoef_A_p  period=1  ownership=Locally owned and initialized  arith_type="Signed  (2's comp)"  n_bits=32  bin_pt=31  dbl_ovrd=off  block_type=fromreg
      settings: AttributesFormatString=<< %<shared_memory_name> >>  infoedit="Register block that reads data to a shared memory register.  Delay of one sample period."  block_version=VER_STRING_GOES_HERE
  Phase Adj : Outport
  tag scope: PilotPhaseErr=global
  wires:
  Rx_FreqOffFilt_A_KP/1 -> Down Sample1/1
  Phase Err/1 -> Gateway Out1/1, Mult1/2, [PilotPhaseErr]
  Gateway Out2/1 -> MIMO CFO Loop Filt/1
  Gateway Out1/1 -> MIMO CFO Loop Filt/2
  Rst/1 -> Register1/2
  Vin/1 -> Gateway Out2/1, Delay1/1
  Down Sample1/1 -> Mult1/1
  Register1/1 -> Phase Adj/1, Gateway Out6/1
  Delay1/1 -> Register1/3
  Mult1/1 -> Register1/1
  Gateway Out6/1 -> MIMO CFO Loop Filt/3

== system ofdm_txrx_alamouti/OFDM Rx Alamouti/Equalizer & Packetizer/Loop Filter B ==
  Phase Err : Inport
  Rst : Inport  Port=2
  Vin : Inport  Port=3
  Accumulator : xbsIndex_r4/Accumulator  operation=Add  n_bits=32  overflow=Wrap  scale=1  rst=on  hasbypass=off  en=on  dbl_ovrd=off  use_behavioral_HDL=on  block_type=accum
      settings: infoedit="Adder or subtractor-based accumulator.   Output type and binary point position match the input.<P><P>Hardware notes: When \"Reinitialize with input 'b' on reset\" is selected, the accumulator is forced to run at the system rate even if the input 'b' is running at a slower rate."  block_version=VER_STRING_GOES_HERE
  AddSub : xbsIndex_r4/AddSub  mode=Addition  use_carryin=off  use_carryout=off  en=off  latency=0  precision=User Defined  arith_type="Signed  (2's comp)"  n_bits=32  bin_pt=31  quantization=Truncate  overflow=Wrap  dbl_ovrd=off  use_behavioral_HDL=off  pipelined=off  use_rpm=on  block_type=addsub
      settings: block_version=VER_STRING_GOES_HERE
  Delay : xbsIndex_r4/Delay  en=off  latency=3  dbl_ovrd=off  reg_retiming=off  block_type=delay
      settings: infoedit=Hardware notes: A delay line is a chain, each link of which is an SRL16 followed by a flip-flop. If register retiming is enabled, the delay line is a chain of flip-flops.  block_version=VER_STRING_GOES_HERE
  Display : Display
  Display1 : Display
  Down Sample1 : xbsIndex_r4/Down Sample  sample_ratio=2  sample_phase="Last Value of Frame  (most efficient)"  en=off  latency=1  dbl_ovrd=off  block_type=dsamp
      settings: block_version=VER_STRING_GOES_HERE
  Down Sample2 : xbsIndex_r4/Down Sample  sample_ratio=2  sample_phase="Last Value of Frame  (most efficient)"  en=off  latency=1  dbl_ovrd=off  block_type=dsamp
      settings: block_version=VER_STRING_GOES_HERE
  Gateway Out{1..6} (6 alike) : xbsIndex_r4/Gateway Out  hdl_port=off  timing_constraint=None  locs_specified=off  LOCs={}  block_type=gatewayout
      settings: infoedit="Gateway out block.  Converts Xilinx fixed point inputs into ouputs of type Simulink integer, double, or fixed point.<P><P>Hardware notes:  In hardware these blocks become top level output ports or are discarded, depending on how they are configured."  block_version=VER_STRING_GOES_HERE
  MIMO CFO Loop Filt : Scope  NumInputPorts=6
      settings: YMin=-3.5~-1.575e-006~-1.25e-005~-1.5e-005~0~-1  YMax=2.5~-1.375e-006~1e-005~1e-005~1~1  SaveName=ScopeData40
  Mult : xbsIndex_r4/Mult  precision=User Defined  arith_type="Signed  (2's comp)"  n_bits=32  bin_pt=31  quantization=Truncate  overflow=Wrap  en=off  latency=3  dbl_ovrd=off  use_behavioral_HDL=off  use_embedded=on  opt=Speed  optimum_pipeline=off  use_rpm=off  placement_style=Rectangular shape  pipeline=on  block_type=mult
      settings: infoedit=Hardware notes: To use the internal pipeline stage of the dedicated multiplier you must select 'Pipeline for maximum performance'.  block_version=VER_STRING_GOES_HERE
  Mult1 : xbsIndex_r4/Mult  precision=User Defined  arith_type="Signed  (2's comp)"  n_bits=32  bin_pt=31  quantization=Truncate  overflow=Wrap  en=off  latency=3  dbl_ovrd=off  use_behavioral_HDL=off  use_embedded=on  opt=Speed  optimum_pipeline=off  use_rpm=off  placement_style=Rectangular shape  pipeline=on  block_type=mult
      settings: infoedit=Hardware notes: To use the internal pipeline stage of the dedicated multiplier you must select 'Pipeline for maximum performance'.  block_version=VER_STRING_GOES_HERE
  Rx_FreqOffFilt_KI : xbsIndex_r4/From Register  shared_memory_name='Rx_FreqOffFilt_B_KI'  init=freqOffset_track_filtCoef_i  period=1  ownership=Locally owned and initialized  arith_type=Unsigned  n_bits=32  bin_pt=32  dbl_ovrd=off  block_type=fromreg
      settings: AttributesFormatString=<< %<shared_memory_name> >>  infoedit="Register block that reads data to a shared memory register.  Delay of one sample period."  block_version=VER_STRING_GOES_HERE
  Rx_FreqOffFilt_KP : xbsIndex_r4/From Register  shared_memory_name='Rx_FreqOffFilt_B_KP'  init=freqOffset_track_filtCoef_p  period=1  ownership=Locally owned and initialized  arith_type=Unsigned  n_bits=32  bin_pt=32  dbl_ovrd=off  block_type=fromreg
      settings: AttributesFormatString=<< %<shared_memory_name> >>  infoedit="Register block that reads data to a shared memory register.  Delay of one sample period."  block_version=VER_STRING_GOES_HERE
  Fine Freq : Outport
  wires:
  Rx_FreqOffFilt_KI/1 -> Down Sample2/1
  Mult/1 -> Accumulator/1
  Accumulator/1 -> AddSub/1, Gateway Out1/1
  Rx_FreqOffFilt_KP/1 -> Down Sample1/1
  Phase Err/1 -> Mult1/2, Mult/1, Gateway Out2/1
  Mult1/1 -> AddSub/2, Gateway Out3/1
  AddSub/1 -> Gateway Out4/1, Fine Freq/1
  Gateway Out2/1 -> MIMO CFO Loop Filt/1
  Gateway Out1/1 -> MIMO CFO Loop Filt/2
  Gateway Out3/1 -> MIMO CFO Loop Filt/3
  Gateway Out4/1 -> MIMO CFO Loop Filt/4
  Rst/1 -> Accumulator/2, Gateway Out6/1
  Vin/1 -> Delay/1, Gateway Out5/1
  Delay/1 -> Accumulator/3
  Gateway Out5/1 -> MIMO CFO Loop Filt/5
  Gateway Out6/1 -> MIMO CFO Loop Filt/6
  Down Sample1/1 -> Mult1/1, Display/1
  Down Sample2/1 -> Mult/2, Display1/1
